simulink library "Garbage"  (R2023a) (continued)
== system Garbage/Text_Display_1x16/Character_Table/Characters(G..W)/Char_I ==
  Byte_01 : Constant  Value=c.char(2, c.I)  OutDataTypeStr=uint8
  Byte_02 : Constant  Value=c.char(3, c.I)  OutDataTypeStr=uint8
  Byte_03 : Constant  Value=c.char(4, c.I)  OutDataTypeStr=uint8
  Byte_04 : Constant  Value=c.char(5, c.I)  OutDataTypeStr=uint8
  Byte_05 : Constant  Value=c.char(6, c.I)  OutDataTypeStr=uint8
  Byte_06 : Constant  Value=c.char(7, c.I)  OutDataTypeStr=uint8
  Byte_07 : Constant  Value=c.char(8, c.I)  OutDataTypeStr=uint8
  Byte_1 : Constant  Value=c.char(1, c.I)  OutDataTypeStr=uint8
  Row_7(1B) : Outport
  Row_6(1B) : Outport  Port=2
  Row_5(1B) : Outport  Port=3
  Row_4(1B) : Outport  Port=4
  Row_3(1B) : Outport  Port=5
  Row_2(1B) : Outport  Port=6
  Row_1(1B) : Outport  Port=7
  Row_0(1B) : Outport  Port=8
  wires:
  Byte_02/1 -> Row_5(1B)/1
  Byte_1/1 -> Row_7(1B)/1
  Byte_06/1 -> Row_1(1B)/1
  Byte_03/1 -> Row_4(1B)/1
  Byte_07/1 -> Row_0(1B)/1
  Byte_01/1 -> Row_6(1B)/1
  Byte_04/1 -> Row_3(1B)/1
  Byte_05/1 -> Row_2(1B)/1

== system Garbage/Text_Display_1x16/Character_Table/Characters(G..W)/Char_J ==
  Byte_01 : Constant  Value=c.char(2, c.J)  OutDataTypeStr=uint8
  Byte_02 : Constant  Value=c.char(3, c.J)  OutDataTypeStr=uint8
  Byte_03 : Constant  Value=c.char(4, c.J)  OutDataTypeStr=uint8
  Byte_04 : Constant  Value=c.char(5, c.J)  OutDataTypeStr=uint8
  Byte_05 : Constant  Value=c.char(6, c.J)  OutDataTypeStr=uint8
  Byte_06 : Constant  Value=c.char(7, c.J)  OutDataTypeStr=uint8
  Byte_07 : Constant  Value=c.char(8, c.J)  OutDataTypeStr=uint8
  Byte_1 : Constant  Value=c.char(1, c.J)  OutDataTypeStr=uint8
  Row_7(1B) : Outport
  Row_6(1B) : Outport  Port=2
  Row_5(1B) : Outport  Port=3
  Row_4(1B) : Outport  Port=4
  Row_3(1B) : Outport  Port=5
  Row_2(1B) : Outport  Port=6
  Row_1(1B) : Outport  Port=7
  Row_0(1B) : Outport  Port=8
  wires:
  Byte_02/1 -> Row_5(1B)/1
  Byte_1/1 -> Row_7(1B)/1
  Byte_06/1 -> Row_1(1B)/1
  Byte_03/1 -> Row_4(1B)/1
  Byte_07/1 -> Row_0(1B)/1
  Byte_01/1 -> Row_6(1B)/1
  Byte_04/1 -> Row_3(1B)/1
  Byte_05/1 -> Row_2(1B)/1

== system Garbage/Text_Display_1x16/Character_Table/Characters(G..W)/Char_K ==
  Byte_01 : Constant  Value=c.char(2, c.K)  OutDataTypeStr=uint8
  Byte_02 : Constant  Value=c.char(3, c.K)  OutDataTypeStr=uint8
  Byte_03 : Constant  Value=c.char(4, c.K)  OutDataTypeStr=uint8
  Byte_04 : Constant  Value=c.char(5, c.K)  OutDataTypeStr=uint8
  Byte_05 : Constant  Value=c.char(6, c.K)  OutDataTypeStr=uint8
  Byte_06 : Constant  Value=c.char(7, c.K)  OutDataTypeStr=uint8
  Byte_07 : Constant  Value=c.char(8, c.K)  OutDataTypeStr=uint8
  Byte_1 : Constant  Value=c.char(1, c.K)  OutDataTypeStr=uint8
  Row_7(1B) : Outport
  Row_6(1B) : Outport  Port=2
  Row_5(1B) : Outport  Port=3
  Row_4(1B) : Outport  Port=4
  Row_3(1B) : Outport  Port=5
  Row_2(1B) : Outport  Port=6
  Row_1(1B) : Outport  Port=7
  Row_0(1B) : Outport  Port=8
  wires:
  Byte_02/1 -> Row_5(1B)/1
  Byte_1/1 -> Row_7(1B)/1
  Byte_06/1 -> Row_1(1B)/1
  Byte_03/1 -> Row_4(1B)/1
  Byte_07/1 -> Row_0(1B)/1
  Byte_01/1 -> Row_6(1B)/1
  Byte_04/1 -> Row_3(1B)/1
  Byte_05/1 -> Row_2(1B)/1

== system Garbage/Text_Display_1x16/Character_Table/Characters(G..W)/Char_L ==
  Byte_01 : Constant  Value=c.char(2, c.L)  OutDataTypeStr=uint8
  Byte_02 : Constant  Value=c.char(3, c.L)  OutDataTypeStr=uint8
  Byte_03 : Constant  Value=c.char(4, c.L)  OutDataTypeStr=uint8
  Byte_04 : Constant  Value=c.char(5, c.L)  OutDataTypeStr=uint8
  Byte_05 : Constant  Value=c.char(6, c.L)  OutDataTypeStr=uint8
  Byte_06 : Constant  Value=c.char(7, c.L)  OutDataTypeStr=uint8
  Byte_07 : Constant  Value=c.char(8, c.L)  OutDataTypeStr=uint8
  Byte_1 : Constant  Value=c.char(1, c.L)  OutDataTypeStr=uint8
  Row_7(1B) : Outport
  Row_6(1B) : Outport  Port=2
  Row_5(1B) : Outport  Port=3
  Row_4(1B) : Outport  Port=4
  Row_3(1B) : Outport  Port=5
  Row_2(1B) : Outport  Port=6
  Row_1(1B) : Outport  Port=7
  Row_0(1B) : Outport  Port=8
  wires:
  Byte_02/1 -> Row_5(1B)/1
  Byte_1/1 -> Row_7(1B)/1
  Byte_06/1 -> Row_1(1B)/1
  Byte_03/1 -> Row_4(1B)/1
  Byte_07/1 -> Row_0(1B)/1
  Byte_01/1 -> Row_6(1B)/1
  Byte_04/1 -> Row_3(1B)/1
  Byte_05/1 -> Row_2(1B)/1

== system Garbage/Text_Display_1x16/Character_Table/Characters(G..W)/Char_M ==
  Byte_01 : Constant  Value=c.char(2, c.M)  OutDataTypeStr=uint8
  Byte_02 : Constant  Value=c.char(3, c.M)  OutDataTypeStr=uint8
  Byte_03 : Constant  Value=c.char(4, c.M)  OutDataTypeStr=uint8
  Byte_04 : Constant  Value=c.char(5, c.M)  OutDataTypeStr=uint8
  Byte_05 : Constant  Value=c.char(6, c.M)  OutDataTypeStr=uint8
  Byte_06 : Constant  Value=c.char(7, c.M)  OutDataTypeStr=uint8
  Byte_07 : Constant  Value=c.char(8, c.M)  OutDataTypeStr=uint8
  Byte_1 : Constant  Value=c.char(1, c.M)  OutDataTypeStr=uint8
  Row_7(1B) : Outport
  Row_6(1B) : Outport  Port=2
  Row_5(1B) : Outport  Port=3
  Row_4(1B) : Outport  Port=4
  Row_3(1B) : Outport  Port=5
  Row_2(1B) : Outport  Port=6
  Row_1(1B) : Outport  Port=7
  Row_0(1B) : Outport  Port=8
  wires:
  Byte_02/1 -> Row_5(1B)/1
  Byte_1/1 -> Row_7(1B)/1
  Byte_06/1 -> Row_1(1B)/1
  Byte_03/1 -> Row_4(1B)/1
  Byte_07/1 -> Row_0(1B)/1
  Byte_01/1 -> Row_6(1B)/1
  Byte_04/1 -> Row_3(1B)/1
  Byte_05/1 -> Row_2(1B)/1

== system Garbage/Text_Display_1x16/Character_Table/Characters(G..W)/Char_N ==
  Byte_01 : Constant  Value=c.char(2, c.N)  OutDataTypeStr=uint8
  Byte_02 : Constant  Value=c.char(3, c.N)  OutDataTypeStr=uint8
  Byte_03 : Constant  Value=c.char(4, c.N)  OutDataTypeStr=uint8
  Byte_04 : Constant  Value=c.char(5, c.N)  OutDataTypeStr=uint8
  Byte_05 : Constant  Value=c.char(6, c.N)  OutDataTypeStr=uint8
  Byte_06 : Constant  Value=c.char(7, c.N)  OutDataTypeStr=uint8
  Byte_07 : Constant  Value=c.char(8, c.N)  OutDataTypeStr=uint8
  Byte_1 : Constant  Value=c.char(1, c.N)  OutDataTypeStr=uint8
  Row_7(1B) : Outport
  Row_6(1B) : Outport  Port=2
  Row_5(1B) : Outport  Port=3
  Row_4(1B) : Outport  Port=4
  Row_3(1B) : Outport  Port=5
  Row_2(1B) : Outport  Port=6
  Row_1(1B) : Outport  Port=7
  Row_0(1B) : Outport  Port=8
  wires:
  Byte_02/1 -> Row_5(1B)/1
  Byte_1/1 -> Row_7(1B)/1
  Byte_06/1 -> Row_1(1B)/1
  Byte_03/1 -> Row_4(1B)/1
  Byte_07/1 -> Row_0(1B)/1
  Byte_01/1 -> Row_6(1B)/1
  Byte_04/1 -> Row_3(1B)/1
  Byte_05/1 -> Row_2(1B)/1

== system Garbage/Text_Display_1x16/Character_Table/Characters(G..W)/Char_O ==
  Byte_01 : Constant  Value=c.char(2, c.O)  OutDataTypeStr=uint8
  Byte_02 : Constant  Value=c.char(3, c.O)  OutDataTypeStr=uint8
  Byte_03 : Constant  Value=c.char(4, c.O)  OutDataTypeStr=uint8
  Byte_04 : Constant  Value=c.char(5, c.O)  OutDataTypeStr=uint8
  Byte_05 : Constant  Value=c.char(6, c.O)  OutDataTypeStr=uint8
  Byte_06 : Constant  Value=c.char(7, c.O)  OutDataTypeStr=uint8
  Byte_07 : Constant  Value=c.char(8, c.O)  OutDataTypeStr=uint8
  Byte_1 : Constant  Value=c.char(1, c.O)  OutDataTypeStr=uint8
  Row_7(1B) : Outport
  Row_6(1B) : Outport  Port=2
  Row_5(1B) : Outport  Port=3
  Row_4(1B) : Outport  Port=4
  Row_3(1B) : Outport  Port=5
  Row_2(1B) : Outport  Port=6
  Row_1(1B) : Outport  Port=7
  Row_0(1B) : Outport  Port=8
  wires:
  Byte_02/1 -> Row_5(1B)/1
  Byte_1/1 -> Row_7(1B)/1
  Byte_06/1 -> Row_1(1B)/1
  Byte_03/1 -> Row_4(1B)/1
  Byte_07/1 -> Row_0(1B)/1
  Byte_01/1 -> Row_6(1B)/1
  Byte_04/1 -> Row_3(1B)/1
  Byte_05/1 -> Row_2(1B)/1

== system Garbage/Text_Display_1x16/Character_Table/Characters(G..W)/Char_P ==
  Byte_01 : Constant  Value=c.char(2, c.P)  OutDataTypeStr=uint8
  Byte_02 : Constant  Value=c.char(3, c.P)  OutDataTypeStr=uint8
  Byte_03 : Constant  Value=c.char(4, c.P)  OutDataTypeStr=uint8
  Byte_04 : Constant  Value=c.char(5, c.P)  OutDataTypeStr=uint8
  Byte_05 : Constant  Value=c.char(6, c.P)  OutDataTypeStr=uint8
  Byte_06 : Constant  Value=c.char(7, c.P)  OutDataTypeStr=uint8
  Byte_07 : Constant  Value=c.char(8, c.P)  OutDataTypeStr=uint8
  Byte_1 : Constant  Value=c.char(1, c.P)  OutDataTypeStr=uint8
  Row_7(1B) : Outport
  Row_6(1B) : Outport  Port=2
  Row_5(1B) : Outport  Port=3
  Row_4(1B) : Outport  Port=4
  Row_3(1B) : Outport  Port=5
  Row_2(1B) : Outport  Port=6
  Row_1(1B) : Outport  Port=7
  Row_0(1B) : Outport  Port=8
  wires:
  Byte_02/1 -> Row_5(1B)/1
  Byte_1/1 -> Row_7(1B)/1
  Byte_06/1 -> Row_1(1B)/1
  Byte_03/1 -> Row_4(1B)/1
  Byte_07/1 -> Row_0(1B)/1
  Byte_01/1 -> Row_6(1B)/1
  Byte_04/1 -> Row_3(1B)/1
  Byte_05/1 -> Row_2(1B)/1

== system Garbage/Text_Display_1x16/Character_Table/Characters(G..W)/Char_R ==
  Byte_01 : Constant  Value=c.char(2, c.R)  OutDataTypeStr=uint8
  Byte_02 : Constant  Value=c.char(3, c.R)  OutDataTypeStr=uint8
  Byte_03 : Constant  Value=c.char(4, c.R)  OutDataTypeStr=uint8
  Byte_04 : Constant  Value=c.char(5, c.R)  OutDataTypeStr=uint8
  Byte_05 : Constant  Value=c.char(6, c.R)  OutDataTypeStr=uint8
  Byte_06 : Constant  Value=c.char(7, c.R)  OutDataTypeStr=uint8
  Byte_07 : Constant  Value=c.char(8, c.R)  OutDataTypeStr=uint8
  Byte_1 : Constant  Value=c.char(1, c.R)  OutDataTypeStr=uint8
  Row_7(1B) : Outport
  Row_6(1B) : Outport  Port=2
  Row_5(1B) : Outport  Port=3
  Row_4(1B) : Outport  Port=4
  Row_3(1B) : Outport  Port=5
  Row_2(1B) : Outport  Port=6
  Row_1(1B) : Outport  Port=7
  Row_0(1B) : Outport  Port=8
  wires:
  Byte_02/1 -> Row_5(1B)/1
  Byte_1/1 -> Row_7(1B)/1
  Byte_06/1 -> Row_1(1B)/1
  Byte_03/1 -> Row_4(1B)/1
  Byte_07/1 -> Row_0(1B)/1
  Byte_01/1 -> Row_6(1B)/1
  Byte_04/1 -> Row_3(1B)/1
  Byte_05/1 -> Row_2(1B)/1

== system Garbage/Text_Display_1x16/Character_Table/Characters(G..W)/Char_S ==
  Byte_01 : Constant  Value=c.char(2, c.S)  OutDataTypeStr=uint8
  Byte_02 : Constant  Value=c.char(3, c.S)  OutDataTypeStr=uint8
  Byte_03 : Constant  Value=c.char(4, c.S)  OutDataTypeStr=uint8
  Byte_04 : Constant  Value=c.char(5, c.S)  OutDataTypeStr=uint8
  Byte_05 : Constant  Value=c.char(6, c.S)  OutDataTypeStr=uint8
  Byte_06 : Constant  Value=c.char(7, c.S)  OutDataTypeStr=uint8
  Byte_07 : Constant  Value=c.char(8, c.S)  OutDataTypeStr=uint8
  Byte_1 : Constant  Value=c.char(1, c.S)  OutDataTypeStr=uint8
  Row_7(1B) : Outport
  Row_6(1B) : Outport  Port=2
  Row_5(1B) : Outport  Port=3
  Row_4(1B) : Outport  Port=4
  Row_3(1B) : Outport  Port=5
  Row_2(1B) : Outport  Port=6
  Row_1(1B) : Outport  Port=7
  Row_0(1B) : Outport  Port=8
  wires:
  Byte_02/1 -> Row_5(1B)/1
  Byte_1/1 -> Row_7(1B)/1
  Byte_06/1 -> Row_1(1B)/1
  Byte_03/1 -> Row_4(1B)/1
  Byte_07/1 -> Row_0(1B)/1
  Byte_01/1 -> Row_6(1B)/1
  Byte_04/1 -> Row_3(1B)/1
  Byte_05/1 -> Row_2(1B)/1

== system Garbage/Text_Display_1x16/Character_Table/Characters(G..W)/Char_T ==
  Byte_01 : Constant  Value=c.char(2, c.T)  OutDataTypeStr=uint8
  Byte_02 : Constant  Value=c.char(3, c.T)  OutDataTypeStr=uint8
  Byte_03 : Constant  Value=c.char(4, c.T)  OutDataTypeStr=uint8
  Byte_04 : Constant  Value=c.char(5, c.T)  OutDataTypeStr=uint8
  Byte_05 : Constant  Value=c.char(6, c.T)  OutDataTypeStr=uint8
  Byte_06 : Constant  Value=c.char(7, c.T)  OutDataTypeStr=uint8
  Byte_07 : Constant  Value=c.char(8, c.T)  OutDataTypeStr=uint8
  Byte_1 : Constant  Value=c.char(1, c.T)  OutDataTypeStr=uint8
  Row_7(1B) : Outport
  Row_6(1B) : Outport  Port=2
  Row_5(1B) : Outport  Port=3
  Row_4(1B) : Outport  Port=4
  Row_3(1B) : Outport  Port=5
  Row_2(1B) : Outport  Port=6
  Row_1(1B) : Outport  Port=7
  Row_0(1B) : Outport  Port=8
  wires:
  Byte_02/1 -> Row_5(1B)/1
  Byte_1/1 -> Row_7(1B)/1
  Byte_06/1 -> Row_1(1B)/1
  Byte_03/1 -> Row_4(1B)/1
  Byte_07/1 -> Row_0(1B)/1
  Byte_01/1 -> Row_6(1B)/1
  Byte_04/1 -> Row_3(1B)/1
  Byte_05/1 -> Row_2(1B)/1

== system Garbage/Text_Display_1x16/Character_Table/Characters(G..W)/Char_U ==
  Byte_01 : Constant  Value=c.char(2, c.U)  OutDataTypeStr=uint8
  Byte_02 : Constant  Value=c.char(3, c.U)  OutDataTypeStr=uint8
  Byte_03 : Constant  Value=c.char(4, c.U)  OutDataTypeStr=uint8
  Byte_04 : Constant  Value=c.char(5, c.U)  OutDataTypeStr=uint8
  Byte_05 : Constant  Value=c.char(6, c.U)  OutDataTypeStr=uint8
  Byte_06 : Constant  Value=c.char(7, c.U)  OutDataTypeStr=uint8
  Byte_07 : Constant  Value=c.char(8, c.U)  OutDataTypeStr=uint8
  Byte_1 : Constant  Value=c.char(1, c.U)  OutDataTypeStr=uint8
  Row_7(1B) : Outport
  Row_6(1B) : Outport  Port=2
  Row_5(1B) : Outport  Port=3
  Row_4(1B) : Outport  Port=4
  Row_3(1B) : Outport  Port=5
  Row_2(1B) : Outport  Port=6
  Row_1(1B) : Outport  Port=7
  Row_0(1B) : Outport  Port=8
  wires:
  Byte_02/1 -> Row_5(1B)/1
  Byte_1/1 -> Row_7(1B)/1
  Byte_06/1 -> Row_1(1B)/1
  Byte_03/1 -> Row_4(1B)/1
  Byte_07/1 -> Row_0(1B)/1
  Byte_01/1 -> Row_6(1B)/1
  Byte_04/1 -> Row_3(1B)/1
  Byte_05/1 -> Row_2(1B)/1

== system Garbage/Text_Display_1x16/Character_Table/Characters(G..W)/Char_V ==
  Byte_01 : Constant  Value=c.char(2, c.V)  OutDataTypeStr=uint8
  Byte_02 : Constant  Value=c.char(3, c.V)  OutDataTypeStr=uint8
  Byte_03 : Constant  Value=c.char(4, c.V)  OutDataTypeStr=uint8
  Byte_04 : Constant  Value=c.char(5, c.V)  OutDataTypeStr=uint8
  Byte_05 : Constant  Value=c.char(6, c.V)  OutDataTypeStr=uint8
  Byte_06 : Constant  Value=c.char(7, c.V)  OutDataTypeStr=uint8
  Byte_07 : Constant  Value=c.char(8, c.V)  OutDataTypeStr=uint8
  Byte_1 : Constant  Value=c.char(1, c.V)  OutDataTypeStr=uint8
  Row_7(1B) : Outport
  Row_6(1B) : Outport  Port=2
  Row_5(1B) : Outport  Port=3
  Row_4(1B) : Outport  Port=4
  Row_3(1B) : Outport  Port=5
  Row_2(1B) : Outport  Port=6
  Row_1(1B) : Outport  Port=7
  Row_0(1B) : Outport  Port=8
  wires:
  Byte_02/1 -> Row_5(1B)/1
  Byte_1/1 -> Row_7(1B)/1
  Byte_06/1 -> Row_1(1B)/1
  Byte_03/1 -> Row_4(1B)/1
  Byte_07/1 -> Row_0(1B)/1
  Byte_01/1 -> Row_6(1B)/1
  Byte_04/1 -> Row_3(1B)/1
  Byte_05/1 -> Row_2(1B)/1

== system Garbage/Text_Display_1x16/Character_Table/Characters(G..W)/Char_W ==
  Byte_01 : Constant  Value=c.char(2, c.W)  OutDataTypeStr=uint8
  Byte_02 : Constant  Value=c.char(3, c.W)  OutDataTypeStr=uint8
  Byte_03 : Constant  Value=c.char(4, c.W)  OutDataTypeStr=uint8
  Byte_04 : Constant  Value=c.char(5, c.W)  OutDataTypeStr=uint8
  Byte_05 : Constant  Value=c.char(6, c.W)  OutDataTypeStr=uint8
  Byte_06 : Constant  Value=c.char(7, c.W)  OutDataTypeStr=uint8
  Byte_07 : Constant  Value=c.char(8, c.W)  OutDataTypeStr=uint8
  Byte_1 : Constant  Value=c.char(1, c.W)  OutDataTypeStr=uint8
  Row_7(1B) : Outport
  Row_6(1B) : Outport  Port=2
  Row_5(1B) : Outport  Port=3
  Row_4(1B) : Outport  Port=4
  Row_3(1B) : Outport  Port=5
  Row_2(1B) : Outport  Port=6
  Row_1(1B) : Outport  Port=7
  Row_0(1B) : Outport  Port=8
  wires:
  Byte_02/1 -> Row_5(1B)/1
  Byte_1/1 -> Row_7(1B)/1
  Byte_06/1 -> Row_1(1B)/1
  Byte_03/1 -> Row_4(1B)/1
  Byte_07/1 -> Row_0(1B)/1
  Byte_01/1 -> Row_6(1B)/1
  Byte_04/1 -> Row_3(1B)/1
  Byte_05/1 -> Row_2(1B)/1

== system Garbage/Text_Display_1x16/Character_Table/Characters(Q..ARROW_UP)/Char_ARROW_UP ==
  Byte_01 : Constant  Value=c.char(2, c.ARROW_UP)  OutDataTypeStr=uint8
  Byte_02 : Constant  Value=c.char(3, c.ARROW_UP)  OutDataTypeStr=uint8
  Byte_03 : Constant  Value=c.char(4, c.ARROW_UP)  OutDataTypeStr=uint8
  Byte_04 : Constant  Value=c.char(5, c.ARROW_UP)  OutDataTypeStr=uint8
  Byte_05 : Constant  Value=c.char(6, c.ARROW_UP)  OutDataTypeStr=uint8
  Byte_06 : Constant  Value=c.char(7, c.ARROW_UP)  OutDataTypeStr=uint8
  Byte_07 : Constant  Value=c.char(8, c.ARROW_UP)  OutDataTypeStr=uint8
  Byte_1 : Constant  Value=c.char(1, c.ARROW_UP)  OutDataTypeStr=uint8
  Row_7(1B) : Outport
  Row_6(1B) : Outport  Port=2
  Row_5(1B) : Outport  Port=3
  Row_4(1B) : Outport  Port=4
  Row_3(1B) : Outport  Port=5
  Row_2(1B) : Outport  Port=6
  Row_1(1B) : Outport  Port=7
  Row_0(1B) : Outport  Port=8
  wires:
  Byte_02/1 -> Row_5(1B)/1
  Byte_1/1 -> Row_7(1B)/1
  Byte_06/1 -> Row_1(1B)/1
  Byte_03/1 -> Row_4(1B)/1
  Byte_07/1 -> Row_0(1B)/1
  Byte_01/1 -> Row_6(1B)/1
  Byte_04/1 -> Row_3(1B)/1
  Byte_05/1 -> Row_2(1B)/1

== system Garbage/Text_Display_1x16/Character_Table/Characters(Q..ARROW_UP)/Char_ASTERISK ==
  Byte_01 : Constant  Value=c.char(2, c.ASTERISK)  OutDataTypeStr=uint8
  Byte_02 : Constant  Value=c.char(3, c.ASTERISK)  OutDataTypeStr=uint8
  Byte_03 : Constant  Value=c.char(4, c.ASTERISK)  OutDataTypeStr=uint8
  Byte_04 : Constant  Value=c.char(5, c.ASTERISK)  OutDataTypeStr=uint8
  Byte_05 : Constant  Value=c.char(6, c.ASTERISK)  OutDataTypeStr=uint8
  Byte_06 : Constant  Value=c.char(7, c.ASTERISK)  OutDataTypeStr=uint8
  Byte_07 : Constant  Value=c.char(8, c.ASTERISK)  OutDataTypeStr=uint8
  Byte_1 : Constant  Value=c.char(1, c.ASTERISK)  OutDataTypeStr=uint8
  Row_7(1B) : Outport
  Row_6(1B) : Outport  Port=2
  Row_5(1B) : Outport  Port=3
  Row_4(1B) : Outport  Port=4
  Row_3(1B) : Outport  Port=5
  Row_2(1B) : Outport  Port=6
  Row_1(1B) : Outport  Port=7
  Row_0(1B) : Outport  Port=8
  wires:
  Byte_02/1 -> Row_5(1B)/1
  Byte_1/1 -> Row_7(1B)/1
  Byte_06/1 -> Row_1(1B)/1
  Byte_03/1 -> Row_4(1B)/1
  Byte_07/1 -> Row_0(1B)/1
  Byte_01/1 -> Row_6(1B)/1
  Byte_04/1 -> Row_3(1B)/1
  Byte_05/1 -> Row_2(1B)/1

== system Garbage/Text_Display_1x16/Character_Table/Characters(Q..ARROW_UP)/Char_BACKSLASH ==
  Byte_01 : Constant  Value=c.char(2, c.BACKSLASH)  OutDataTypeStr=uint8
  Byte_02 : Constant  Value=c.char(3, c.BACKSLASH)  OutDataTypeStr=uint8
  Byte_03 : Constant  Value=c.char(4, c.BACKSLASH)  OutDataTypeStr=uint8
  Byte_04 : Constant  Value=c.char(5, c.BACKSLASH)  OutDataTypeStr=uint8
  Byte_05 : Constant  Value=c.char(6, c.BACKSLASH)  OutDataTypeStr=uint8
  Byte_06 : Constant  Value=c.char(7, c.BACKSLASH)  OutDataTypeStr=uint8
  Byte_07 : Constant  Value=c.char(8, c.BACKSLASH)  OutDataTypeStr=uint8
  Byte_1 : Constant  Value=c.char(1, c.BACKSLASH)  OutDataTypeStr=uint8
  Row_7(1B) : Outport
  Row_6(1B) : Outport  Port=2
  Row_5(1B) : Outport  Port=3
  Row_4(1B) : Outport  Port=4
  Row_3(1B) : Outport  Port=5
  Row_2(1B) : Outport  Port=6
  Row_1(1B) : Outport  Port=7
  Row_0(1B) : Outport  Port=8
  wires:
  Byte_02/1 -> Row_5(1B)/1
  Byte_1/1 -> Row_7(1B)/1
  Byte_06/1 -> Row_1(1B)/1
  Byte_03/1 -> Row_4(1B)/1
  Byte_07/1 -> Row_0(1B)/1
  Byte_01/1 -> Row_6(1B)/1
  Byte_04/1 -> Row_3(1B)/1
  Byte_05/1 -> Row_2(1B)/1

== system Garbage/Text_Display_1x16/Character_Table/Characters(Q..ARROW_UP)/Char_COLON ==
  Byte_01 : Constant  Value=c.char(2, c.COLON)  OutDataTypeStr=uint8
  Byte_02 : Constant  Value=c.char(3, c.COLON)  OutDataTypeStr=uint8
  Byte_03 : Constant  Value=c.char(4, c.COLON)  OutDataTypeStr=uint8
  Byte_04 : Constant  Value=c.char(5, c.COLON)  OutDataTypeStr=uint8
  Byte_05 : Constant  Value=c.char(6, c.COLON)  OutDataTypeStr=uint8
  Byte_06 : Constant  Value=c.char(7, c.COLON)  OutDataTypeStr=uint8
  Byte_07 : Constant  Value=c.char(8, c.COLON)  OutDataTypeStr=uint8
  Byte_1 : Constant  Value=c.char(1, c.COLON)  OutDataTypeStr=uint8
  Row_7(1B) : Outport
  Row_6(1B) : Outport  Port=2
  Row_5(1B) : Outport  Port=3
  Row_4(1B) : Outport  Port=4
  Row_3(1B) : Outport  Port=5
  Row_2(1B) : Outport  Port=6
  Row_1(1B) : Outport  Port=7
  Row_0(1B) : Outport  Port=8
  wires:
  Byte_02/1 -> Row_5(1B)/1
  Byte_1/1 -> Row_7(1B)/1
  Byte_06/1 -> Row_1(1B)/1
  Byte_03/1 -> Row_4(1B)/1
  Byte_07/1 -> Row_0(1B)/1
  Byte_01/1 -> Row_6(1B)/1
  Byte_04/1 -> Row_3(1B)/1
  Byte_05/1 -> Row_2(1B)/1

== system Garbage/Text_Display_1x16/Character_Table/Characters(Q..ARROW_UP)/Char_CROSS ==
  Byte_01 : Constant  Value=c.char(2, c.CROSS)  OutDataTypeStr=uint8
  Byte_02 : Constant  Value=c.char(3, c.CROSS)  OutDataTypeStr=uint8
  Byte_03 : Constant  Value=c.char(4, c.CROSS)  OutDataTypeStr=uint8
  Byte_04 : Constant  Value=c.char(5, c.CROSS)  OutDataTypeStr=uint8
  Byte_05 : Constant  Value=c.char(6, c.CROSS)  OutDataTypeStr=uint8
  Byte_06 : Constant  Value=c.char(7, c.CROSS)  OutDataTypeStr=uint8
  Byte_07 : Constant  Value=c.char(8, c.CROSS)  OutDataTypeStr=uint8
  Byte_1 : Constant  Value=c.char(1, c.CROSS)  OutDataTypeStr=uint8
  Row_7(1B) : Outport
  Row_6(1B) : Outport  Port=2
  Row_5(1B) : Outport  Port=3
  Row_4(1B) : Outport  Port=4
  Row_3(1B) : Outport  Port=5
  Row_2(1B) : Outport  Port=6
  Row_1(1B) : Outport  Port=7
  Row_0(1B) : Outport  Port=8
  wires:
  Byte_02/1 -> Row_5(1B)/1
  Byte_1/1 -> Row_7(1B)/1
  Byte_06/1 -> Row_1(1B)/1
  Byte_03/1 -> Row_4(1B)/1
  Byte_07/1 -> Row_0(1B)/1
  Byte_01/1 -> Row_6(1B)/1
  Byte_04/1 -> Row_3(1B)/1
  Byte_05/1 -> Row_2(1B)/1

== system Garbage/Text_Display_1x16/Character_Table/Characters(Q..ARROW_UP)/Char_EQUAL ==
  Byte_01 : Constant  Value=c.char(2, c.EQUAL)  OutDataTypeStr=uint8
  Byte_02 : Constant  Value=c.char(3, c.EQUAL)  OutDataTypeStr=uint8
  Byte_03 : Constant  Value=c.char(4, c.EQUAL)  OutDataTypeStr=uint8
  Byte_04 : Constant  Value=c.char(5, c.EQUAL)  OutDataTypeStr=uint8
  Byte_05 : Constant  Value=c.char(6, c.EQUAL)  OutDataTypeStr=uint8
  Byte_06 : Constant  Value=c.char(7, c.EQUAL)  OutDataTypeStr=uint8
  Byte_07 : Constant  Value=c.char(8, c.EQUAL)  OutDataTypeStr=uint8
  Byte_1 : Constant  Value=c.char(1, c.EQUAL)  OutDataTypeStr=uint8
  Row_7(1B) : Outport
  Row_6(1B) : Outport  Port=2
  Row_5(1B) : Outport  Port=3
  Row_4(1B) : Outport  Port=4
  Row_3(1B) : Outport  Port=5
  Row_2(1B) : Outport  Port=6
  Row_1(1B) : Outport  Port=7
  Row_0(1B) : Outport  Port=8
  wires:
  Byte_02/1 -> Row_5(1B)/1
  Byte_1/1 -> Row_7(1B)/1
  Byte_06/1 -> Row_1(1B)/1
  Byte_03/1 -> Row_4(1B)/1
  Byte_07/1 -> Row_0(1B)/1
  Byte_01/1 -> Row_6(1B)/1
  Byte_04/1 -> Row_3(1B)/1
  Byte_05/1 -> Row_2(1B)/1

== system Garbage/Text_Display_1x16/Character_Table/Characters(Q..ARROW_UP)/Char_GREATER_THAN ==
  Byte_01 : Constant  Value=c.char(2, c.GREATER_THAN)  OutDataTypeStr=uint8
  Byte_02 : Constant  Value=c.char(3, c.GREATER_THAN)  OutDataTypeStr=uint8
  Byte_03 : Constant  Value=c.char(4, c.GREATER_THAN)  OutDataTypeStr=uint8
  Byte_04 : Constant  Value=c.char(5, c.GREATER_THAN)  OutDataTypeStr=uint8
  Byte_05 : Constant  Value=c.char(6, c.GREATER_THAN)  OutDataTypeStr=uint8
  Byte_06 : Constant  Value=c.char(7, c.GREATER_THAN)  OutDataTypeStr=uint8
  Byte_07 : Constant  Value=c.char(8, c.GREATER_THAN)  OutDataTypeStr=uint8
  Byte_1 : Constant  Value=c.char(1, c.GREATER_THAN)  OutDataTypeStr=uint8
  Row_7(1B) : Outport
  Row_6(1B) : Outport  Port=2
  Row_5(1B) : Outport  Port=3
  Row_4(1B) : Outport  Port=4
  Row_3(1B) : Outport  Port=5
  Row_2(1B) : Outport  Port=6
  Row_1(1B) : Outport  Port=7
  Row_0(1B) : Outport  Port=8
  wires:
  Byte_02/1 -> Row_5(1B)/1
  Byte_1/1 -> Row_7(1B)/1
  Byte_06/1 -> Row_1(1B)/1
  Byte_03/1 -> Row_4(1B)/1
  Byte_07/1 -> Row_0(1B)/1
  Byte_01/1 -> Row_6(1B)/1
  Byte_04/1 -> Row_3(1B)/1
  Byte_05/1 -> Row_2(1B)/1

== system Garbage/Text_Display_1x16/Character_Table/Characters(Q..ARROW_UP)/Char_LESS_THAN ==
  Byte_01 : Constant  Value=c.char(2, c.LESS_THAN)  OutDataTypeStr=uint8
  Byte_02 : Constant  Value=c.char(3, c.LESS_THAN)  OutDataTypeStr=uint8
  Byte_03 : Constant  Value=c.char(4, c.LESS_THAN)  OutDataTypeStr=uint8
  Byte_04 : Constant  Value=c.char(5, c.LESS_THAN)  OutDataTypeStr=uint8
  Byte_05 : Constant  Value=c.char(6, c.LESS_THAN)  OutDataTypeStr=uint8
  Byte_06 : Constant  Value=c.char(7, c.LESS_THAN)  OutDataTypeStr=uint8
  Byte_07 : Constant  Value=c.char(8, c.LESS_THAN)  OutDataTypeStr=uint8
  Byte_1 : Constant  Value=c.char(1, c.LESS_THAN)  OutDataTypeStr=uint8
  Row_7(1B) : Outport
  Row_6(1B) : Outport  Port=2
  Row_5(1B) : Outport  Port=3
  Row_4(1B) : Outport  Port=4
  Row_3(1B) : Outport  Port=5
  Row_2(1B) : Outport  Port=6
  Row_1(1B) : Outport  Port=7
  Row_0(1B) : Outport  Port=8
  wires:
  Byte_02/1 -> Row_5(1B)/1
  Byte_1/1 -> Row_7(1B)/1
  Byte_06/1 -> Row_1(1B)/1
  Byte_03/1 -> Row_4(1B)/1
  Byte_07/1 -> Row_0(1B)/1
  Byte_01/1 -> Row_6(1B)/1
  Byte_04/1 -> Row_3(1B)/1
  Byte_05/1 -> Row_2(1B)/1

== system Garbage/Text_Display_1x16/Character_Table/Characters(Q..ARROW_UP)/Char_MINUS ==
  Byte_01 : Constant  Value=c.char(2, c.MINUS)  OutDataTypeStr=uint8
  Byte_02 : Constant  Value=c.char(3, c.MINUS)  OutDataTypeStr=uint8
  Byte_03 : Constant  Value=c.char(4, c.MINUS)  OutDataTypeStr=uint8
  Byte_04 : Constant  Value=c.char(5, c.MINUS)  OutDataTypeStr=uint8
  Byte_05 : Constant  Value=c.char(6, c.MINUS)  OutDataTypeStr=uint8
  Byte_06 : Constant  Value=c.char(7, c.MINUS)  OutDataTypeStr=uint8
  Byte_07 : Constant  Value=c.char(8, c.MINUS)  OutDataTypeStr=uint8
  Byte_1 : Constant  Value=c.char(1, c.MINUS)  OutDataTypeStr=uint8
  Row_7(1B) : Outport
  Row_6(1B) : Outport  Port=2
  Row_5(1B) : Outport  Port=3
  Row_4(1B) : Outport  Port=4
  Row_3(1B) : Outport  Port=5
  Row_2(1B) : Outport  Port=6
  Row_1(1B) : Outport  Port=7
  Row_0(1B) : Outport  Port=8
  wires:
  Byte_02/1 -> Row_5(1B)/1
  Byte_1/1 -> Row_7(1B)/1
  Byte_06/1 -> Row_1(1B)/1
  Byte_03/1 -> Row_4(1B)/1
  Byte_07/1 -> Row_0(1B)/1
  Byte_01/1 -> Row_6(1B)/1
  Byte_04/1 -> Row_3(1B)/1
  Byte_05/1 -> Row_2(1B)/1
